simulink model "MX_Gateway"  (Simulink 9.0)
settings: Solver=FixedStepDiscrete  StartTime=0.0  StopTime=100  FixedStep=0.01  RelTol=1e-3
values from neighbouring files (symbols this model uses):
  ACTIVE = Bobcat.Parameter; CreateDefine( ACTIVE                         ,1                          ,'Define' ,'defines.h' )    [defines.m]
  ACTIVE_MODE_ONE = Bobcat.Parameter; CreateDefine( ACTIVE_MODE_ONE                ,19                         ,'Define' ,'defines.h' )    [defines.m]
  AUTHENTICATED = Bobcat.Parameter; CreateDefine( AUTHENTICATED                  ,1                          ,'Define' ,'defines.h' )    [defines.m]
  AUX_EMEA_CONTROLS = Bobcat.Parameter; CreateDefine( AUX_EMEA_CONTROLS  ,1   ,'Define' ,'defines.h' )    [enums.m]
  AUX_FLOW_HIGH = Bobcat.Parameter; CreateDefine( AUX_FLOW_HIGH           ,4  ,'Define' ,'defines.h' )    [enums.m]
  AUX_FLOW_INACTIVE = Bobcat.Parameter; CreateDefine( AUX_FLOW_INACTIVE       ,0  ,'Define' ,'defines.h' )    [enums.m]
  AUX_FLOW_LOW = Bobcat.Parameter; CreateDefine( AUX_FLOW_LOW            ,1  ,'Define' ,'defines.h' )    [enums.m]
  AUX_FLOW_MEDIUM = Bobcat.Parameter; CreateDefine( AUX_FLOW_MEDIUM         ,2  ,'Define' ,'defines.h' )    [enums.m]
  AUX_FLOW_STANDARD = Bobcat.Parameter; CreateDefine( AUX_FLOW_STANDARD       ,3  ,'Define' ,'defines.h' )    [enums.m]
  AUX_NON_FUNCTIONAL = Bobcat.Parameter; CreateDefine( AUX_NON_FUNCTIONAL ,255 ,'Define' ,'defines.h' )    [enums.m]
  BASE_ACTIVE = Bobcat.Parameter; CreateDefine( BASE_ACTIVE                    ,253                        ,'Define' ,'defines.h' )    [defines.m]
  BASE_DETENT = Bobcat.Parameter; CreateDefine( BASE_DETENT                    ,1                          ,'Define' ,'defines.h' )    [defines.m]
  BEEP_NONE = Bobcat.Parameter; CreateDefine( BEEP_NONE                      ,255                        ,'Define' ,'defines.h' )    [defines.m]
  CD_AIR_FILTER_DEBOUNCE_NORMAL = Bobcat.Parameter; CreateAliasCD( CD_AIR_FILTER_DEBOUNCE_NORMAL                        ,uint16(500)                ,'ConstantDataPointer' ,'CONSTANT_DATA' ,'AIR_FILTER_DEBOUNCE_NORMAL')    [cdata.m]    (also in hc12_cdata.m; m4_cdata.m)
  CD_AIR_FILTER_DEBOUNCE_NOT_CONNECTED = Bobcat.Parameter; CreateAliasCD( CD_AIR_FILTER_DEBOUNCE_NOT_CONNECTED                 ,uint16(3000)               ,'ConstantDataPointer' ,'CONSTANT_DATA' ,'AIR_FILTER_DEBOUNCE_NOT_CONNECTED')    [cdata.m]    (also in hc12_cdata.m; m4_cdata.m)
  CD_AIR_FILTER_DEBOUNCE_PLUGGED = Bobcat.Parameter; CreateAliasCD( CD_AIR_FILTER_DEBOUNCE_PLUGGED                       ,uint16(3000)               ,'ConstantDataPointer' ,'CONSTANT_DATA' ,'AIR_FILTER_DEBOUNCE_PLUGGED')    [cdata.m]    (also in hc12_cdata.m; m4_cdata.m)
  CD_AIR_FILTER_FUNCTION = Bobcat.Parameter; CreateAliasCD( CD_AIR_FILTER_FUNCTION                               ,uint8(255)                 ,'ConstantDataPointer' ,'CONSTANT_DATA' ,'AIR_FILTER_FUNCTION')    [cdata.m]    (also in hc12_cdata.m; m4_cdata.m)
  CD_AIR_FILTER_PLUGGED_WEIGHT = Bobcat.Parameter; CreateAliasCD( CD_AIR_FILTER_PLUGGED_WEIGHT                         ,uint8(2)                   ,'ConstantDataPointer' ,'CONSTANT_DATA' ,'AIR_FILTER_PLUGGED_WEIGHT')    [cdata.m]    (also in hc12_cdata.m; m4_cdata.m)
  CD_AIR_FILTER_UNPLUGGED_WEIGHT = Bobcat.Parameter; CreateAliasCD( CD_AIR_FILTER_UNPLUGGED_WEIGHT                       ,uint8(1)                   ,'ConstantDataPointer' ,'CONSTANT_DATA' ,'AIR_FILTER_UNPLUGGED_WEIGHT')    [cdata.m]    (also in hc12_cdata.m; m4_cdata.m)
  CD_AUTOIDLE_ARMBAR_DELAY = Bobcat.Parameter; CreateAliasCD( CD_AUTOIDLE_ARMBAR_DELAY                             ,uint8(2)                   ,'ConstantDataPointer' ,'CONSTANT_DATA' ,'AUTOIDLE_ARMBAR_DELAY')    [cdata.m]    (also in hc12_cdata.m; m4_cdata.m)
  CD_AUTOIDLE_FUNCTION = Bobcat.Parameter; CreateAliasCD( CD_AUTOIDLE_FUNCTION                                 ,uint8(255)                 ,'ConstantDataPointer' ,'CONSTANT_DATA' ,'AUTOIDLE_FUNCTION')    [cdata.m]    (also in hc12_cdata.m; m4_cdata.m)
  CD_AUTOIDLE_IDLE_DELAY = Bobcat.Parameter; CreateAliasCD( CD_AUTOIDLE_IDLE_DELAY                               ,uint8(40)                  ,'ConstantDataPointer' ,'CONSTANT_DATA' ,'AUTOIDLE_IDLE_DELAY')    [cdata.m]    (also in hc12_cdata.m; m4_cdata.m)
  CD_AUTOIDLE_LOW_IDLE_START = Bobcat.Parameter; CreateAliasCD( CD_AUTOIDLE_LOW_IDLE_START                           ,uint8(255)                 ,'ConstantDataPointer' ,'CONSTANT_DATA' ,'AUTOIDLE_LOW_IDLE_START')    [cdata.m]    (also in hc12_cdata.m; m4_cdata.m)
  CD_AUTOIDLE_PRESSURE_OFF = Bobcat.Parameter; CreateAliasCD( CD_AUTOIDLE_PRESSURE_OFF                             ,uint16(300)                ,'ConstantDataPointer' ,'CONSTANT_DATA' ,'AUTOIDLE_PRESSURE_OFF')    [cdata.m]    (also in hc12_cdata.m; m4_cdata.m)
  CD_AUTOIDLE_PRESSURE_ON = Bobcat.Parameter; CreateAliasCD( CD_AUTOIDLE_PRESSURE_ON                              ,uint16(270)                ,'ConstantDataPointer' ,'CONSTANT_DATA' ,'AUTOIDLE_PRESSURE_ON')    [cdata.m]    (also in hc12_cdata.m; m4_cdata.m)
  CD_AUTOSHIFT_FUNCTION = Bobcat.Parameter; CreateAliasCD( CD_AUTOSHIFT_FUNCTION                                ,uint8(255)                 ,'ConstantDataPointer' ,'CONSTANT_DATA' ,'AUTOSHIFT_FUNCTION')    [cdata.m]    (also in hc12_cdata.m; m4_cdata.m)
  CD_AUTOSHIFT_PHIGH1 = Bobcat.Parameter; CreateAliasCD( CD_AUTOSHIFT_PHIGH1                                  ,uint16(205)                ,'ConstantDataPointer' ,'CONSTANT_DATA' ,'AUTOSHIFT_PHIGH1')    [cdata.m]    (also in hc12_cdata.m; m4_cdata.m)
  CD_AUTOSHIFT_PHIGH2 = Bobcat.Parameter; CreateAliasCD( CD_AUTOSHIFT_PHIGH2                                  ,uint16(193)                ,'ConstantDataPointer' ,'CONSTANT_DATA' ,'AUTOSHIFT_PHIGH2')    [cdata.m]    (also in hc12_cdata.m; m4_cdata.m)
  CD_AUTOSHIFT_PHIGH_TEMP1 = Bobcat.Parameter; CreateAliasCD( CD_AUTOSHIFT_PHIGH_TEMP1                             ,int16(70)                  ,'ConstantDataPointer' ,'CONSTANT_DATA' ,'AUTOSHIFT_PHIGH_TEMP1')    [cdata.m]    (also in hc12_cdata.m; m4_cdata.m)
  CD_AUTOSHIFT_PHIGH_TEMP2 = Bobcat.Parameter; CreateAliasCD( CD_AUTOSHIFT_PHIGH_TEMP2                             ,int16(160)                 ,'ConstantDataPointer' ,'CONSTANT_DATA' ,'AUTOSHIFT_PHIGH_TEMP2')    [cdata.m]    (also in hc12_cdata.m; m4_cdata.m)
  CD_AUTOSHIFT_PLOW1 = Bobcat.Parameter; CreateAliasCD( CD_AUTOSHIFT_PLOW1                                   ,uint16(130)                ,'ConstantDataPointer' ,'CONSTANT_DATA' ,'AUTOSHIFT_PLOW1')    [cdata.m]    (also in hc12_cdata.m; m4_cdata.m)
  CD_AUTOSHIFT_PLOW2 = Bobcat.Parameter; CreateAliasCD( CD_AUTOSHIFT_PLOW2                                   ,uint16(100)                ,'ConstantDataPointer' ,'CONSTANT_DATA' ,'AUTOSHIFT_PLOW2')    [cdata.m]    (also in hc12_cdata.m; m4_cdata.m)
  CD_AUTOSHIFT_PLOW_TEMP1 = Bobcat.Parameter; CreateAliasCD( CD_AUTOSHIFT_PLOW_TEMP1                              ,int16(20)                  ,'ConstantDataPointer' ,'CONSTANT_DATA' ,'AUTOSHIFT_PLOW_TEMP1')    [cdata.m]    (also in hc12_cdata.m; m4_cdata.m)
  CD_AUTOSHIFT_PLOW_TEMP2 = Bobcat.Parameter; CreateAliasCD( CD_AUTOSHIFT_PLOW_TEMP2                              ,int16(80)                  ,'ConstantDataPointer' ,'CONSTANT_DATA' ,'AUTOSHIFT_PLOW_TEMP2')    [cdata.m]    (also in hc12_cdata.m; m4_cdata.m)
  CD_AUTOSHIFT_TRAVEL_DELAY = Bobcat.Parameter; CreateAliasCD( CD_AUTOSHIFT_TRAVEL_DELAY                            ,uint8(32)                  ,'ConstantDataPointer' ,'CONSTANT_DATA' ,'AUTOSHIFT_TRAVEL_DELAY')    [cdata.m]    (also in hc12_cdata.m; m4_cdata.m)
  CD_AUX2_CRACKPOINT_CURRENT = Bobcat.Parameter; CreateAliasCD( CD_AUX2_CRACKPOINT_CURRENT                           ,fi(0,0,16,12,'hex','0c28') ,'ConstantDataPointer' ,'CONSTANT_DATA' ,'AUX2_CRACKPOINT_CURRENT')    [cdata.m]    (also in hc12_cdata.m; m4_cdata.m)
  CD_AUX2_FLOW_LOW_FUNCTION = Bobcat.Parameter; CreateAliasCD( CD_AUX2_FLOW_LOW_FUNCTION                            ,uint8(255)                 ,'ConstantDataPointer' ,'CONSTANT_DATA' ,'AUX2_FLOW_LOW_FUNCTION')    [cdata.m]    (also in hc12_cdata.m; m4_cdata.m)
  CD_AUX2_FLOW_MED_FUNCTION = Bobcat.Parameter; CreateAliasCD( CD_AUX2_FLOW_MED_FUNCTION                            ,uint8(255)                 ,'ConstantDataPointer' ,'CONSTANT_DATA' ,'AUX2_FLOW_MED_FUNCTION')    [cdata.m]    (also in hc12_cdata.m; m4_cdata.m)
  CD_AUX2_HI_FLOW_PERCENT = Bobcat.Parameter; CreateAliasCD( CD_AUX2_HI_FLOW_PERCENT                              ,fi(0,0,16,16,'hex','ffff') ,'ConstantDataPointer' ,'CONSTANT_DATA' ,'AUX2_HI_FLOW_PERCENT')    [cdata.m]    (also in hc12_cdata.m; m4_cdata.m)
  CD_AUX2_LOW_FLOW_PERCENT = Bobcat.Parameter; CreateAliasCD( CD_AUX2_LOW_FLOW_PERCENT                             ,fi(0,0,16,16,'hex','7333') ,'ConstantDataPointer' ,'CONSTANT_DATA' ,'AUX2_LOW_FLOW_PERCENT')    [cdata.m]    (also in hc12_cdata.m; m4_cdata.m)
  CD_AUX2_MAX_FLOW_CURRENT = Bobcat.Parameter; CreateAliasCD( CD_AUX2_MAX_FLOW_CURRENT                             ,fi(0,0,16,12,'hex','14cc') ,'ConstantDataPointer' ,'CONSTANT_DATA' ,'AUX2_MAX_FLOW_CURRENT')    [cdata.m]    (also in hc12_cdata.m; m4_cdata.m)
  CD_AUX2_MED_FLOW_PERCENT = Bobcat.Parameter; CreateAliasCD( CD_AUX2_MED_FLOW_PERCENT                             ,fi(0,0,16,16,'hex','9999') ,'ConstantDataPointer' ,'CONSTANT_DATA' ,'AUX2_MED_FLOW_PERCENT')    [cdata.m]    (also in hc12_cdata.m; m4_cdata.m)
  CD_AUX_CRACKPOINT_CURRENT = Bobcat.Parameter; CreateAliasCD( CD_AUX_CRACKPOINT_CURRENT                            ,fi(0,0,16,12,'hex','0000') ,'ConstantDataPointer' ,'CONSTANT_DATA' ,'AUX_CRACKPOINT_CURRENT')    [cdata.m]    (also in hc12_cdata.m; m4_cdata.m)
  CD_AUX_DETENT_FLASH_TIME = Bobcat.Parameter; CreateAliasCD( CD_AUX_DETENT_FLASH_TIME                             ,uint16(72)                 ,'ConstantDataPointer' ,'CONSTANT_DATA' ,'AUX_DETENT_FLASH_TIME')    [cdata.m]    (also in hc12_cdata.m; m4_cdata.m)
  CD_AUX_DETENT_HOLD_TIME = Bobcat.Parameter; CreateAliasCD( CD_AUX_DETENT_HOLD_TIME                              ,uint16(100)                ,'ConstantDataPointer' ,'CONSTANT_DATA' ,'AUX_DETENT_HOLD_TIME')    [cdata.m]    (also in hc12_cdata.m; m4_cdata.m)
  CD_AUX_FULL_ON_AUX1 = Bobcat.Parameter; CreateAliasCD( CD_AUX_FULL_ON_AUX1                                  ,fi(0,0,16,12,'hex','10cd') ,'ConstantDataPointer' ,'CONSTANT_DATA' ,'AUX_FULL_ON_AUX1')    [cdata.m]    (also in hc12_cdata.m; m4_cdata.m)
  CD_AUX_FULL_ON_AUX2 = Bobcat.Parameter; CreateAliasCD( CD_AUX_FULL_ON_AUX2                                  ,fi(0,0,16,12,'hex','139d') ,'ConstantDataPointer' ,'CONSTANT_DATA' ,'AUX_FULL_ON_AUX2')    [cdata.m]    (also in hc12_cdata.m; m4_cdata.m)
  CD_AUX_FULL_ON_AUX3 = Bobcat.Parameter; CreateAliasCD( CD_AUX_FULL_ON_AUX3                                  ,fi(0,0,16,12,'hex','17d7') ,'ConstantDataPointer' ,'CONSTANT_DATA' ,'AUX_FULL_ON_AUX3')    [cdata.m]    (also in hc12_cdata.m; m4_cdata.m)
  CD_AUX_FUNCTION = Bobcat.Parameter; CreateAliasCD( CD_AUX_FUNCTION                                      ,uint8(0)                   ,'ConstantDataPointer' ,'CONSTANT_DATA' ,'AUX_FUNCTION')    [cdata.m]    (also in hc12_cdata.m; m4_cdata.m)
  CD_AUX_RATE_LIMIT_CRACKPOINT = Bobcat.Parameter; CreateAliasCD( CD_AUX_RATE_LIMIT_CRACKPOINT                         ,fi(0,0,16,12,'hex','0800') ,'ConstantDataPointer' ,'CONSTANT_DATA' ,'AUX_RATE_LIMIT_CRACKPOINT')    [cdata.m]    (also in hc12_cdata.m; m4_cdata.m)
  CD_AUX_RATE_LIMIT_CRACKPOINT_RATE = Bobcat.Parameter; CreateAliasCD( CD_AUX_RATE_LIMIT_CRACKPOINT_RATE                    ,fi(0,1,32,12,'int',102400) ,'ConstantDataPointer' ,'CONSTANT_DATA' ,'AUX_RATE_LIMIT_CRACKPOINT_RATE')    [cdata.m]    (also in hc12_cdata.m; m4_cdata.m)
  CD_AUX_RATE_LIMIT_FUNCTION = Bobcat.Parameter; CreateAliasCD( CD_AUX_RATE_LIMIT_FUNCTION                           ,uint8(255)                 ,'ConstantDataPointer' ,'CONSTANT_DATA' ,'AUX_RATE_LIMIT_FUNCTION')    [cdata.m]    (also in hc12_cdata.m; m4_cdata.m)
  CD_AUX_RATE_LIMIT_MAX_ENG_SPD = Bobcat.Parameter; CreateAliasCD( CD_AUX_RATE_LIMIT_MAX_ENG_SPD                        ,uint16(1600)               ,'ConstantDataPointer' ,'CONSTANT_DATA' ,'AUX_RATE_LIMIT_MAX_ENG_SPD')    [cdata.m]    (also in hc12_cdata.m; m4_cdata.m)
  CD_AUX_RATE_LIMIT_MIN_ENG_SPD = Bobcat.Parameter; CreateAliasCD( CD_AUX_RATE_LIMIT_MIN_ENG_SPD                        ,uint16(100)                ,'ConstantDataPointer' ,'CONSTANT_DATA' ,'AUX_RATE_LIMIT_MIN_ENG_SPD')    [cdata.m]    (also in hc12_cdata.m; m4_cdata.m)
  CD_AUX_RATE_LIMIT_NORMAL_RATE = Bobcat.Parameter; CreateAliasCD( CD_AUX_RATE_LIMIT_NORMAL_RATE                        ,fi(0,1,32,12,'int',2048)   ,'ConstantDataPointer' ,'CONSTANT_DATA' ,'AUX_RATE_LIMIT_NORMAL_RATE')    [cdata.m]    (also in hc12_cdata.m; m4_cdata.m)
  CD_AUXPOT_CAL_DEADBAND_HIGH = Bobcat.Parameter; CreateAliasCD( CD_AUXPOT_CAL_DEADBAND_HIGH                          ,uint16(574)                ,'ConstantDataPointer' ,'CONSTANT_DATA' ,'AUXPOT_CAL_DEADBAND_HIGH')    [cdata.m]    (also in hc12_cdata.m; m4_cdata.m)
  CD_AUXPOT_CAL_DEADBAND_LOW = Bobcat.Parameter; CreateAliasCD( CD_AUXPOT_CAL_DEADBAND_LOW                           ,uint16(424)                ,'ConstantDataPointer' ,'CONSTANT_DATA' ,'AUXPOT_CAL_DEADBAND_LOW')    [cdata.m]    (also in hc12_cdata.m; m4_cdata.m)
  CD_AUXPOT_CENTER_RATIO = Bobcat.Parameter; CreateAliasCD( CD_AUXPOT_CENTER_RATIO                               ,uint16(500)                ,'ConstantDataPointer' ,'CONSTANT_DATA' ,'AUXPOT_CENTER_RATIO')    [cdata.m]    (also in hc12_cdata.m; m4_cdata.m)
  CD_AUXPOT_DEADBAND = Bobcat.Parameter; CreateAliasCD( CD_AUXPOT_DEADBAND                                   ,uint8(38)                  ,'ConstantDataPointer' ,'CONSTANT_DATA' ,'AUXPOT_DEADBAND')    [cdata.m]    (also in hc12_cdata.m; m4_cdata.m)
  CD_AUXPOT_FUNCTION = Bobcat.Parameter; CreateAliasCD( CD_AUXPOT_FUNCTION                                   ,uint8(0)                   ,'ConstantDataPointer' ,'CONSTANT_DATA' ,'AUXPOT_FUNCTION')    [cdata.m]    (also in hc12_cdata.m; m4_cdata.m)
  CD_AUXPOT_RATIO_GAIN = Bobcat.Parameter; CreateAliasCD( CD_AUXPOT_RATIO_GAIN                                 ,uint16(1000)               ,'ConstantDataPointer' ,'CONSTANT_DATA' ,'AUXPOT_RATIO_GAIN')    [cdata.m]    (also in hc12_cdata.m; m4_cdata.m)
  CD_CAN_THROTTLE_FUNCTION = Bobcat.Parameter; CreateAliasCD( CD_CAN_THROTTLE_FUNCTION                             ,uint8(255)                 ,'ConstantDataPointer' ,'CONSTANT_DATA' ,'CAN_THROTTLE_FUNCTION')    [cdata.m]    (also in hc12_cdata.m; m4_cdata.m)
  CD_CAN_THROTTLE_REVERSE = Bobcat.Parameter; CreateAliasCD( CD_CAN_THROTTLE_REVERSE                              ,uint8(255)                 ,'ConstantDataPointer' ,'CONSTANT_DATA' ,'CAN_THROTTLE_REVERSE')    [cdata.m]    (also in hc12_cdata.m; m4_cdata.m)
  CD_CONSOLE_DEBOUNCE_ABSENT = Bobcat.Parameter; CreateAliasCD( CD_CONSOLE_DEBOUNCE_ABSENT                           ,uint16(12)                 ,'ConstantDataPointer' ,'CONSTANT_DATA' ,'CONSOLE_DEBOUNCE_ABSENT')    [cdata.m]    (also in hc12_cdata.m; m4_cdata.m)
  CD_CONSOLE_DEBOUNCE_OORH = Bobcat.Parameter; CreateAliasCD( CD_CONSOLE_DEBOUNCE_OORH                             ,uint16(12)                 ,'ConstantDataPointer' ,'CONSTANT_DATA' ,'CONSOLE_DEBOUNCE_OORH')    [cdata.m]    (also in hc12_cdata.m; m4_cdata.m)
  CD_CONSOLE_DEBOUNCE_OORL = Bobcat.Parameter; CreateAliasCD( CD_CONSOLE_DEBOUNCE_OORL                             ,uint16(12)                 ,'ConstantDataPointer' ,'CONSTANT_DATA' ,'CONSOLE_DEBOUNCE_OORL')    [cdata.m]    (also in hc12_cdata.m; m4_cdata.m)
  CD_CONSOLE_DEBOUNCE_PRESENT = Bobcat.Parameter; CreateAliasCD( CD_CONSOLE_DEBOUNCE_PRESENT                          ,uint16(12)                 ,'ConstantDataPointer' ,'CONSTANT_DATA' ,'CONSOLE_DEBOUNCE_PRESENT')    [cdata.m]    (also in hc12_cdata.m; m4_cdata.m)
  CD_CONSOLE_FUNCTION = Bobcat.Parameter; CreateAliasCD( CD_CONSOLE_FUNCTION                                  ,uint8(0)                   ,'ConstantDataPointer' ,'CONSTANT_DATA' ,'CONSOLE_FUNCTION')    [cdata.m]    (also in hc12_cdata.m; m4_cdata.m)
  CD_CONSOLE_OORH = Bobcat.Parameter; CreateAliasCD( CD_CONSOLE_OORH                                      ,uint16(740)                ,'ConstantDataPointer' ,'CONSTANT_DATA' ,'CONSOLE_OORH')    [cdata.m]    (also in hc12_cdata.m; m4_cdata.m)
  CD_CONSOLE_OORL = Bobcat.Parameter; CreateAliasCD( CD_CONSOLE_OORL                                      ,uint16(10)                 ,'ConstantDataPointer' ,'CONSTANT_DATA' ,'CONSOLE_OORL')    [cdata.m]    (also in hc12_cdata.m; m4_cdata.m)
  CD_CONSOLE_PRESENT_HIGH = Bobcat.Parameter; CreateAliasCD( CD_CONSOLE_PRESENT_HIGH                              ,uint16(515)                ,'ConstantDataPointer' ,'CONSTANT_DATA' ,'CONSOLE_PRESENT_HIGH')    [cdata.m]    (also in hc12_cdata.m; m4_cdata.m)
  CD_CONSOLE_PRESENT_LOW = Bobcat.Parameter; CreateAliasCD( CD_CONSOLE_PRESENT_LOW                               ,uint16(375)                ,'ConstantDataPointer' ,'CONSTANT_DATA' ,'CONSOLE_PRESENT_LOW')    [cdata.m]    (also in hc12_cdata.m; m4_cdata.m)
  CD_DELUXE_GEN5_5_FUNCTION = Bobcat.Parameter; CreateAliasCD( CD_DELUXE_GEN5_5_FUNCTION                            ,uint8(255)                 ,'ConstantDataPointer' ,'CONSTANT_DATA' ,'DELUXE_GEN5_5_FUNCTION')    [cdata.m]    (also in hc12_cdata.m; m4_cdata.m)
  CD_DELUXE_GEN5_FUNCTION = Bobcat.Parameter; CreateAliasCD( CD_DELUXE_GEN5_FUNCTION                              ,uint8(255)                 ,'ConstantDataPointer' ,'CONSTANT_DATA' ,'DELUXE_GEN5_FUNCTION')    [cdata.m]    (also in hc12_cdata.m; m4_cdata.m)
  CD_DERATE_ENGINE_SPEED_DEBOUNCE_HIGH = Bobcat.Parameter; CreateAliasCD( CD_DERATE_ENGINE_SPEED_DEBOUNCE_HIGH                 ,uint16(200)                ,'ConstantDataPointer' ,'CONSTANT_DATA' ,'DERATE_ENGINE_SPEED_DEBOUNCE_HIGH')    [cdata.m]    (also in hc12_cdata.m; m4_cdata.m)
  CD_DERATE_ENGINE_SPEED_DEBOUNCE_SHUTDOWN = Bobcat.Parameter; CreateAliasCD( CD_DERATE_ENGINE_SPEED_DEBOUNCE_SHUTDOWN             ,uint16(1000)               ,'ConstantDataPointer' ,'CONSTANT_DATA' ,'DERATE_ENGINE_SPEED_DEBOUNCE_SHUTDOWN')    [cdata.m]    (also in hc12_cdata.m; m4_cdata.m)
  CD_DERATE_ENGINE_SPEED_HIGH_OFFSET = Bobcat.Parameter; CreateAliasCD( CD_DERATE_ENGINE_SPEED_HIGH_OFFSET                   ,uint16(200)                ,'ConstantDataPointer' ,'CONSTANT_DATA' ,'DERATE_ENGINE_SPEED_HIGH_OFFSET')    [cdata.m]    (also in hc12_cdata.m; m4_cdata.m)
  CD_DERATE_METHOD = Bobcat.Parameter; CreateAliasCD( CD_DERATE_METHOD                                     ,uint8(0)                   ,'ConstantDataPointer' ,'CONSTANT_DATA' ,'DERATE_METHOD')    [cdata.m]    (also in hc12_cdata.m; m4_cdata.m)
  CD_DUMP_VALVE_FUNCTION = Bobcat.Parameter; CreateAliasCD( CD_DUMP_VALVE_FUNCTION                               ,uint8(255)                 ,'ConstantDataPointer' ,'CONSTANT_DATA' ,'DUMP_VALVE_FUNCTION')    [cdata.m]    (also in hc12_cdata.m; m4_cdata.m)
  CD_DUMP_VALVE_ON_CURRENT = Bobcat.Parameter; CreateAliasCD( CD_DUMP_VALVE_ON_CURRENT                             ,fi(0,0,16,12,'hex','ffff') ,'ConstantDataPointer' ,'CONSTANT_DATA' ,'DUMP_VALVE_ON_CURRENT')    [cdata.m]    (also in hc12_cdata.m; m4_cdata.m)
  CD_ECO_MODE_THROTTLE_REDUCTION = Bobcat.Parameter; CreateAliasCD( CD_ECO_MODE_THROTTLE_REDUCTION                       ,uint16(850)                ,'ConstantDataPointer' ,'CONSTANT_DATA' ,'ECO_MODE_THROTTLE_REDUCTION')    [cdata.m]    (also in hc12_cdata.m; m4_cdata.m)
  CD_ECT_FILTER_GAIN = Bobcat.Parameter; CreateAliasCD( CD_ECT_FILTER_GAIN                                   ,uint8(6)                   ,'ConstantDataPointer' ,'CONSTANT_DATA' ,'ECT_FILTER_GAIN')    [cdata.m]    (also in hc12_cdata.m; m4_cdata.m)
  CD_ECU_FUNCTION = Bobcat.Parameter; CreateAliasCD( CD_ECU_FUNCTION                                      ,uint8(255)                 ,'ConstantDataPointer' ,'CONSTANT_DATA' ,'ECU_FUNCTION')    [cdata.m]    (also in hc12_cdata.m; m4_cdata.m)
  CD_ECU_HOLD_DEBOUNCE_ERROR_ON = Bobcat.Parameter; CreateAliasCD( CD_ECU_HOLD_DEBOUNCE_ERROR_ON                        ,uint16(20)                 ,'ConstantDataPointer' ,'CONSTANT_DATA' ,'ECU_HOLD_DEBOUNCE_ERROR_ON')    [cdata.m]    (also in hc12_cdata.m; m4_cdata.m)
  CD_EMEA_CONTROLS_HOLD_TIME = Bobcat.Parameter; CreateAliasCD( CD_EMEA_CONTROLS_HOLD_TIME                           ,uint16(30)                 ,'ConstantDataPointer' ,'CONSTANT_DATA' ,'EMEA_CONTROLS_HOLD_TIME')    [cdata.m]    (also in hc12_cdata.m; m4_cdata.m)
  CD_EMEA_CONTROLS_OPTION_A = Bobcat.Parameter; CreateAliasCD( CD_EMEA_CONTROLS_OPTION_A                            ,uint8(255)                 ,'ConstantDataPointer' ,'CONSTANT_DATA' ,'EMEA_CONTROLS_OPTION_A')    [cdata.m]    (also in hc12_cdata.m; m4_cdata.m)
  CD_EMEA_CONTROLS_OPTION_C = Bobcat.Parameter; CreateAliasCD( CD_EMEA_CONTROLS_OPTION_C                            ,uint8(255)                 ,'ConstantDataPointer' ,'CONSTANT_DATA' ,'EMEA_CONTROLS_OPTION_C')    [cdata.m]    (also in hc12_cdata.m; m4_cdata.m)
  CD_EMEA_CONTROLS_REVERSE = Bobcat.Parameter; CreateAliasCD( CD_EMEA_CONTROLS_REVERSE                             ,uint8(255)                 ,'ConstantDataPointer' ,'CONSTANT_DATA' ,'EMEA_CONTROLS_REVERSE')    [cdata.m]    (also in hc12_cdata.m; m4_cdata.m)
  CD_EMEA_ELECTRIC_VERSION = Bobcat.Parameter; CreateAliasCD( CD_EMEA_ELECTRIC_VERSION                             ,uint8(0)                   ,'ConstantDataPointer' ,'CONSTANT_DATA' ,'EMEA_ELECTRIC_VERSION')    [cdata.m]    (also in hc12_cdata.m; m4_cdata.m)
  CD_EMEA_POT_CAL_DEADBAND_HIGH = Bobcat.Parameter; CreateAliasCD( CD_EMEA_POT_CAL_DEADBAND_HIGH                        ,uint16(574)                ,'ConstantDataPointer' ,'CONSTANT_DATA' ,'EMEA_POT_CAL_DEADBAND_HIGH')    [cdata.m]    (also in hc12_cdata.m; m4_cdata.m)
  CD_EMEA_POT_CAL_DEADBAND_LOW = Bobcat.Parameter; CreateAliasCD( CD_EMEA_POT_CAL_DEADBAND_LOW                         ,uint16(424)                ,'ConstantDataPointer' ,'CONSTANT_DATA' ,'EMEA_POT_CAL_DEADBAND_LOW')    [cdata.m]    (also in hc12_cdata.m; m4_cdata.m)
  CD_ENGINE_RPM_FACTOR = Bobcat.Parameter; CreateAliasCD( CD_ENGINE_RPM_FACTOR                                 ,uint8(35)                  ,'ConstantDataPointer' ,'CONSTANT_DATA' ,'ENGINE_RPM_FACTOR')    [cdata.m]    (also in hc12_cdata.m; m4_cdata.m)
  CD_ENGINE_SPEED_FILTER_FAST_VALUE = Bobcat.Parameter; CreateAliasCD( CD_ENGINE_SPEED_FILTER_FAST_VALUE                    ,uint8(1)                   ,'ConstantDataPointer' ,'CONSTANT_DATA' ,'ENGINE_SPEED_FILTER_FAST_VALUE')    [cdata.m]    (also in hc12_cdata.m; m4_cdata.m)
  CD_ENGINE_SPEED_FILTER_SLOW_VALUE = Bobcat.Parameter; CreateAliasCD( CD_ENGINE_SPEED_FILTER_SLOW_VALUE                    ,uint8(4)                   ,'ConstantDataPointer' ,'CONSTANT_DATA' ,'ENGINE_SPEED_FILTER_SLOW_VALUE')    [cdata.m]    (also in hc12_cdata.m; m4_cdata.m)
  CD_ENGINE_SPEED_RUNNING = Bobcat.Parameter; CreateAliasCD( CD_ENGINE_SPEED_RUNNING                              ,uint16(800)                ,'ConstantDataPointer' ,'CONSTANT_DATA' ,'ENGINE_SPEED_RUNNING')    [cdata.m]    (also in hc12_cdata.m; m4_cdata.m)
  CD_ENGINE_START_DELAY_DEBOUNCE_RPM_NO_SIGNAL = Bobcat.Parameter; CreateAliasCD( CD_ENGINE_START_DELAY_DEBOUNCE_RPM_NO_SIGNAL         ,uint16(0)                  ,'ConstantDataPointer' ,'CONSTANT_DATA' ,'ENGINE_START_DELAY_DEBOUNCE_RPM_NO_SIGNAL')    [cdata.m]    (also in hc12_cdata.m; m4_cdata.m)
  CD_ENGINE_START_DELAY_OFF_TIME = Bobcat.Parameter; CreateAliasCD( CD_ENGINE_START_DELAY_OFF_TIME                       ,uint16(0)                  ,'ConstantDataPointer' ,'CONSTANT_DATA' ,'ENGINE_START_DELAY_OFF_TIME')    [cdata.m]    (also in hc12_cdata.m; m4_cdata.m)
  CD_ENGINE_START_DELAY_RPM_NO_SIGNAL = Bobcat.Parameter; CreateAliasCD( CD_ENGINE_START_DELAY_RPM_NO_SIGNAL                  ,uint16(0)                  ,'ConstantDataPointer' ,'CONSTANT_DATA' ,'ENGINE_START_DELAY_RPM_NO_SIGNAL')    [cdata.m]    (also in hc12_cdata.m; m4_cdata.m)
  CD_EOP_DEBOUNCE_EXTREMELY_LOW = Bobcat.Parameter; CreateAliasCD( CD_EOP_DEBOUNCE_EXTREMELY_LOW                        ,uint16(50)                 ,'ConstantDataPointer' ,'CONSTANT_DATA' ,'EOP_DEBOUNCE_EXTREMELY_LOW')    [cdata.m]    (also in hc12_cdata.m; m4_cdata.m)
  CD_EOP_DEBOUNCE_NORMAL = Bobcat.Parameter; CreateAliasCD( CD_EOP_DEBOUNCE_NORMAL                               ,uint16(50)                 ,'ConstantDataPointer' ,'CONSTANT_DATA' ,'EOP_DEBOUNCE_NORMAL')    [cdata.m]    (also in hc12_cdata.m; m4_cdata.m)
  CD_EOP_DEBOUNCE_SHUTDOWN = Bobcat.Parameter; CreateAliasCD( CD_EOP_DEBOUNCE_SHUTDOWN                             ,uint16(100)                ,'ConstantDataPointer' ,'CONSTANT_DATA' ,'EOP_DEBOUNCE_SHUTDOWN')    [cdata.m]    (also in hc12_cdata.m; m4_cdata.m)
  CD_EOP_FILTER_VALUE = Bobcat.Parameter; CreateAliasCD( CD_EOP_FILTER_VALUE                                  ,uint8(3)                   ,'ConstantDataPointer' ,'CONSTANT_DATA' ,'EOP_FILTER_VALUE')    [cdata.m]    (also in hc12_cdata.m; m4_cdata.m)
  CD_EOP_FUNCTION = Bobcat.Parameter; CreateAliasCD( CD_EOP_FUNCTION                                      ,uint8(1)                   ,'ConstantDataPointer' ,'CONSTANT_DATA' ,'EOP_FUNCTION')    [cdata.m]    (also in hc12_cdata.m; m4_cdata.m)
  CD_EOP_SWITCH_GAUGE_VALUE = Bobcat.Parameter; CreateAliasCD( CD_EOP_SWITCH_GAUGE_VALUE                            ,uint16(125)                ,'ConstantDataPointer' ,'CONSTANT_DATA' ,'EOP_SWITCH_GAUGE_VALUE')    [cdata.m]    (also in hc12_cdata.m; m4_cdata.m)
  CD_EOP_SWITCH_LOW = Bobcat.Parameter; CreateAliasCD( CD_EOP_SWITCH_LOW                                    ,uint16(50)                 ,'ConstantDataPointer' ,'CONSTANT_DATA' ,'EOP_SWITCH_LOW')    [cdata.m]    (also in hc12_cdata.m; m4_cdata.m)
  CD_EOP_SWITCH_PRESSURE_VALUE = Bobcat.Parameter; CreateAliasCD( CD_EOP_SWITCH_PRESSURE_VALUE                         ,uint16(40)                 ,'ConstantDataPointer' ,'CONSTANT_DATA' ,'EOP_SWITCH_PRESSURE_VALUE')    [cdata.m]    (also in hc12_cdata.m; m4_cdata.m)
  CD_EOT_DEBOUNCE_EXTREMELY_LOW = Bobcat.Parameter; CreateAliasCD( CD_EOT_DEBOUNCE_EXTREMELY_LOW                        ,uint16(2)                  ,'ConstantDataPointer' ,'CONSTANT_DATA' ,'EOT_DEBOUNCE_EXTREMELY_LOW')    [cdata.m]    (also in hc12_cdata.m; m4_cdata.m)
  CD_EOT_DEBOUNCE_LOW = Bobcat.Parameter; CreateAliasCD( CD_EOT_DEBOUNCE_LOW                                  ,uint16(2)                  ,'ConstantDataPointer' ,'CONSTANT_DATA' ,'EOT_DEBOUNCE_LOW')    [cdata.m]    (also in hc12_cdata.m; m4_cdata.m)
  CD_EOT_DEBOUNCE_NORMAL = Bobcat.Parameter; CreateAliasCD( CD_EOT_DEBOUNCE_NORMAL                               ,uint16(2)                  ,'ConstantDataPointer' ,'CONSTANT_DATA' ,'EOT_DEBOUNCE_NORMAL')    [cdata.m]    (also in hc12_cdata.m; m4_cdata.m)
  CD_EOT_EXTREMELY_LOW = Bobcat.Parameter; CreateAliasCD( CD_EOT_EXTREMELY_LOW                                 ,uint16(81)                 ,'ConstantDataPointer' ,'CONSTANT_DATA' ,'EOT_EXTREMELY_LOW')    [cdata.m]    (also in hc12_cdata.m; m4_cdata.m)
  CD_EOT_FUNCTION = Bobcat.Parameter; CreateAliasCD( CD_EOT_FUNCTION                                      ,uint8(255)                 ,'ConstantDataPointer' ,'CONSTANT_DATA' ,'EOT_FUNCTION')    [cdata.m]    (also in hc12_cdata.m; m4_cdata.m)
  CD_EOT_LOW = Bobcat.Parameter; CreateAliasCD( CD_EOT_LOW                                           ,uint16(90)                 ,'ConstantDataPointer' ,'CONSTANT_DATA' ,'EOT_LOW')    [cdata.m]    (also in hc12_cdata.m; m4_cdata.m)
  CD_EOT_TEMPERATURE_HYSTERESIS = Bobcat.Parameter; CreateAliasCD( CD_EOT_TEMPERATURE_HYSTERESIS                        ,uint16(2)                  ,'ConstantDataPointer' ,'CONSTANT_DATA' ,'EOT_TEMPERATURE_HYSTERESIS')    [cdata.m]    (also in hc12_cdata.m; m4_cdata.m)
  CD_FAST_TRAVEL_FUNCTION = Bobcat.Parameter; CreateAliasCD( CD_FAST_TRAVEL_FUNCTION                              ,uint8(255)                 ,'ConstantDataPointer' ,'CONSTANT_DATA' ,'FAST_TRAVEL_FUNCTION')    [cdata.m]    (also in hc12_cdata.m; m4_cdata.m)
  CD_FAST_TRAVEL_THROTTLE_DELAY = Bobcat.Parameter; CreateAliasCD( CD_FAST_TRAVEL_THROTTLE_DELAY                        ,uint16(10)                 ,'ConstantDataPointer' ,'CONSTANT_DATA' ,'FAST_TRAVEL_THROTTLE_DELAY')    [cdata.m]    (also in hc12_cdata.m; m4_cdata.m)
  CD_FAST_TRAVEL_THROTTLE_LIMIT = Bobcat.Parameter; CreateAliasCD( CD_FAST_TRAVEL_THROTTLE_LIMIT                        ,uint16(1000)               ,'ConstantDataPointer' ,'CONSTANT_DATA' ,'FAST_TRAVEL_THROTTLE_LIMIT')    [cdata.m]    (also in hc12_cdata.m; m4_cdata.m)
  CD_FLYWHEEL_TEETH = Bobcat.Parameter; CreateAliasCD( CD_FLYWHEEL_TEETH                                    ,uint8(83)                  ,'ConstantDataPointer' ,'CONSTANT_DATA' ,'FLYWHEEL_TEETH')    [cdata.m]    (also in hc12_cdata.m; m4_cdata.m)
  CD_FUEL_LEVEL_FILTER_FAST_VALUE = Bobcat.Parameter; CreateAliasCD( CD_FUEL_LEVEL_FILTER_FAST_VALUE                      ,uint8(5)                   ,'ConstantDataPointer' ,'CONSTANT_DATA' ,'FUEL_LEVEL_FILTER_FAST_VALUE')    [cdata.m]    (also in hc12_cdata.m; m4_cdata.m)
  CD_FUEL_LEVEL_FILTER_SLOW_VALUE = Bobcat.Parameter; CreateAliasCD( CD_FUEL_LEVEL_FILTER_SLOW_VALUE                      ,uint8(10)                  ,'ConstantDataPointer' ,'CONSTANT_DATA' ,'FUEL_LEVEL_FILTER_SLOW_VALUE')    [cdata.m]    (also in hc12_cdata.m; m4_cdata.m)
  CD_FUEL_PRIMARY_FEEDBACK_FUNCTION = Bobcat.Parameter; CreateAliasCD( CD_FUEL_PRIMARY_FEEDBACK_FUNCTION                    ,uint8(0)                   ,'ConstantDataPointer' ,'CONSTANT_DATA' ,'FUEL_PRIMARY_FEEDBACK_FUNCTION')    [cdata.m]    (also in hc12_cdata.m; m4_cdata.m)
  ... (+246 more)
undefined symbols (not defined in the file or in its folder): sigbuilder_block
undefined symbols (not defined in the file): CD_FUEL_RESOLUTION_TIMER
libraries: simulink x186, MX_Library x107
(part 18 of 48)

== system MX_Gateway/MX_Gtwy_Control/System/AutoIdle ==
  psi_LoadSense : Inport
  state_LoadSense : Inport  Port=2
  state_Console : Inport  Port=3
  percent_Throttle : Inport  Port=4
  button_AutoIdle : Inport  Port=5
  state_Engine : Inport  Port=6
  state_System : Inport  Port=7
  flag_InitEERead : Inport  Port=8
  dlx_G55_AutoIdleTime : Inport  Port=9
  dlx_G55_AutoIdleEnable : Inport  Port=10
  AutoIdleTime : Truth Table  TreatAsAtomicUnit=on
  Auto_Idle_Enable : Subsystem  RequirementInfo={} %GIDa_1bcaf31e_eccf_4f63_b0fa_03591ea49f6a  TreatAsAtomicUnit=on  SystemSampleTime=srate100  RTWFcnName=mod_engine_control  RTWFileName=mod_System
      settings: RTWSystemCode=Nonreusable function  RTWFcnNameOpts=Use subsystem name  RTWFileNameOpts=User specified
  Auto_Idle_Function : Subsystem
  Compare To Constant : simulink/Logic and Bit Operations/Compare To Constant  relop===  const=SYS_NORMAL  OutDataTypeStr=boolean
  cmd_AutoIdle : Outport  OutputWhenDisabled=reset
  state_AutoIdleEnable : Outport  Port=2  OutputWhenDisabled=reset
  state_Idle : Outport  Port=3
  wires:
  psi_LoadSense/1 -> Auto_Idle_Function/1(psi_LoadSense)
  state_Console/1 -> Auto_Idle_Function/2(state_Console)
  state_Engine/1 -> Auto_Idle_Function/4(state_Engine)
  Auto_Idle_Function/1(cmd_AutoIdle) -> cmd_AutoIdle/1
  percent_Throttle/1 -> Auto_Idle_Function/5(percent_Throttle)
  state_System/1 -> Compare To Constant/1
  Compare To Constant/1 -> Auto_Idle_Function/Enable
  Auto_Idle_Enable/1(state_AutoIdleEnable) -> [state_AutoIdleEnable], state_AutoIdleEnable/1
  state_LoadSense/1 -> Auto_Idle_Enable/2(state_LoadSense)
  button_AutoIdle/1 -> Auto_Idle_Enable/1(button_AutoIdle)
  [state_AutoIdleEnable] -> Auto_Idle_Function/3(state_AutoIdleEnable)
  Auto_Idle_Function/2(state_Idle) -> state_Idle/1
  flag_InitEERead/1 -> Auto_Idle_Enable/3(flag_InitEERead)
  dlx_G55_AutoIdleTime/1 -> AutoIdleTime/1(dlx_G55_AutoIdleTime)
  AutoIdleTime/1(AutoIdleTime) -> Auto_Idle_Function/6(AutoIdleTime)
  dlx_G55_AutoIdleEnable/1 -> Auto_Idle_Enable/4(dlx_G55_AutoIdleEnable)

== system MX_Gateway/MX_Gtwy_Control/System/Aux_Enable ==
  aux_DebounceButton : Inport
  state_AuxPot : Inport  Port=2
  state_console : Inport  Port=3
  aux_DetentHold : Inport  Port=4
  state_Buttons : Inport  Port=5
  button_AuxReleaseAck : Inport  Port=6
  button_AuxReleaseBack : Inport  Port=7
  button_AuxReleaseExit : Inport  Port=8
  button_Aux : Inport  Port=9
  state_RightPot : Inport  Port=10
  state_EngineSpeed : Inport  Port=11
  din_Detent : Inport  Port=12
  state_System : Inport  Port=13
  flag_InitEERead : Inport  Port=14
  state_ST_CAN : Inport  Port=15
  can_AuxEnable_ST : Inport  Port=16
  state_ACD_CAN : Inport  Port=17
  can_AuxEnable_ACD : Inport  Port=18
  state_Engine : Inport  Port=19
  change_OffsetSelectSw : Inport  Port=20
  mode_RightControl : Inport  Port=21
  aux_Inhibit : Inport  Port=22
  Aux_Control : Stateflow chart  RequirementInfo="{}  %GIDa_112d2c1f_2f82_49d8_b987_6745627d4a42"  TreatAsAtomicUnit=on
  DetectFallingEdge : simulink/Logic and Bit Operations/Detect Decrease  vinit=0.0  InputProcessing=Inherited  OutDataTypeStr=uint8
  DetectRisingEdge1 : simulink/Logic and Bit Operations/Detect Increase  vinit=0.0  InputProcessing=Inherited  OutDataTypeStr=uint8
  DetectRisingEdge2 : simulink/Logic and Bit Operations/Detect Increase  vinit=0.0  InputProcessing=Inherited  OutDataTypeStr=uint8
  state_AuxEnable : Outport
  state_Detent : Outport  Port=2
  aux_Flow : Outport  Port=3
  state_DetentEnable : Outport  Port=4
  cmd_AuxRelease : Outport  Port=5
  state_AuxRelease : Outport  Port=6
  wires:
  din_Detent/1 -> DetectRisingEdge1/1
  state_Engine/1 -> Aux_Control/3(state_Engine)
  Aux_Control/1(state_AuxEnable) -> state_AuxEnable/1
  Aux_Control/2(state_Detent) -> state_Detent/1
  state_console/1 -> Aux_Control/4(state_Console)
  DetectRisingEdge1/1 -> Aux_Control/1(DetentRisingEdge)
  Aux_Control/3(aux_Flow) -> aux_Flow/1
  aux_DebounceButton/1 -> Aux_Control/5(aux_DebounceButton)
  Aux_Control/4(state_DetentEnable) -> state_DetentEnable/1
  aux_DetentHold/1 -> DetectRisingEdge2/1
  DetectRisingEdge2/1 -> Aux_Control/7(aux_DetentHold)
  state_Buttons/1 -> Aux_Control/8(state_Buttons)
  state_System/1 -> Aux_Control/6(state_System)
  flag_InitEERead/1 -> Aux_Control/9(flag_InitEERead)
  can_AuxEnable_ST/1 -> Aux_Control/10(can_AuxEnable_ST)
  can_AuxEnable_ACD/1 -> Aux_Control/11(can_AuxEnable_ACD)
  state_ST_CAN/1 -> Aux_Control/12(state_ST_CAN)
  state_ACD_CAN/1 -> Aux_Control/13(state_ACD_CAN)
  Aux_Control/5(cmd_AuxRelease) -> cmd_AuxRelease/1
  button_AuxReleaseAck/1 -> Aux_Control/14(button_AuxReleaseAck)
  button_AuxReleaseBack/1 -> Aux_Control/15(button_AuxReleaseBack)
  button_AuxReleaseExit/1 -> Aux_Control/16(button_AuxReleaseExit)
  button_Aux/1 -> DetectFallingEdge/1
  DetectFallingEdge/1 -> Aux_Control/17(button_Aux)
  Aux_Control/6(state_AuxRelease) -> state_AuxRelease/1
  state_RightPot/1 -> Aux_Control/18(state_RightPot)
  change_OffsetSelectSw/1 -> Aux_Control/19(change_OffsetSelectSw)
  mode_RightControl/1 -> Aux_Control/20(mode_RightControl)
  aux_Inhibit/1 -> Aux_Control/21(aux_Inhibit)
  state_EngineSpeed/1 -> Aux_Control/22(state_EngineSpeed)
  state_AuxPot/1 -> Aux_Control/2(state_AuxPot)

== system MX_Gateway/MX_Gtwy_Control/System/CAN_Throttle ==
  state_System : Inport
  fdbk_CANThrottle : Inport  Port=2
  flag_InitEERead : Inport  Port=3
  pos_Throttle : Inport  Port=4
  state_Engine : Inport  Port=5
  state_CommThrottle : Inport  Port=6
  cmd_Throttle : Inport  Port=7
  CAN_Throttle : Subsystem  TreatAsAtomicUnit=on
  CompareToConstant1 : simulink/Logic and Bit Operations/Compare To Constant  relop=~=  const=NON_FUNCTIONAL  OutDataTypeStr=boolean  ZeroCross=off
  CompareToConstant2 : simulink/Logic and Bit Operations/Compare To Constant  relop===  const=SYS_NORMAL  OutDataTypeStr=boolean  ZeroCross=off
  Constant2 : Constant  Value=CD_CAN_THROTTLE_FUNCTION
  Logical Operator1 : Logic  AllPortsSameDT=off  OutDataTypeStr=boolean
  Unit Delay1 : UnitDelay  SampleTime=-1
      settings: AttributesFormatString=<tsample=%<SampleTime>>\n<initial=%<X0>>
  Unit Delay2 : UnitDelay  SampleTime=-1
      settings: AttributesFormatString=<tsample=%<SampleTime>>\n<initial=%<X0>>
  cmd_CANThrottle : Outport
  state_CANThrottle : Outport  Port=2
  state_CANThrottleCalDiag : Outport  Port=3
  wires:
  state_System/1 -> CompareToConstant2/1
  CompareToConstant1/1 -> Logical Operator1/1
  Constant2/1 -> CompareToConstant1/1
  CompareToConstant2/1 -> Logical Operator1/2
  Logical Operator1/1 -> CAN_Throttle/Enable
  CAN_Throttle/1(cmd_CANThrottle) -> cmd_CANThrottle/1
  fdbk_CANThrottle/1 -> Unit Delay2/1
  flag_InitEERead/1 -> CAN_Throttle/2(flag_InitEERead)
  CAN_Throttle/2(state_CANThrottle) -> state_CANThrottle/1
  pos_Throttle/1 -> CAN_Throttle/3(pos_Throttle)
  state_Engine/1 -> CAN_Throttle/4(state_Engine)
  state_CommThrottle/1 -> CAN_Throttle/5(state_CommThrottle)
  CAN_Throttle/3(state_CANThrottleCalDiag) -> state_CANThrottleCalDiag/1
  cmd_Throttle/1 -> Unit Delay1/1
  Unit Delay1/1 -> CAN_Throttle/6(cmd_Throttle)
  Unit Delay2/1 -> CAN_Throttle/1(fdbk_CANThrottle)

== system MX_Gateway/MX_Gtwy_Control/System/Controller_State ==
  msgAge_Secondary : Inport
  state_System : Inport  Port=2
  msgAge_ECU : Inport  Port=3
  msgAge_Throttle : Inport  Port=4
  msgAge_StdPanel : Inport  Port=5
  msgAge_JogShuttle : Inport  Port=6
  msgAge_DeluxeG55 : Inport  Port=7
  flag_InitEERead : Inport  Port=8
  msgAge_KeylessEntry : Inport  Port=9
  state_Engine : Inport  Port=10
  state_SwitchedPwrOutput : Inport  Port=11
  state_SwitchedPwrRelay : Inport  Port=12
  error_FuelSec : Inport  Port=13
  state_ECUHold : Inport  Port=14
  Controller_State : Stateflow chart  TreatAsAtomicUnit=on
  state_CommSecondary : Outport
  state_CommECU : Outport  Port=2
  state_CommThrottle : Outport  Port=3
  state_CommStdPanel : Outport  Port=4
  state_CommJogShuttle : Outport  Port=5
  state_CommDeluxeG55 : Outport  Port=6
  state_CommKeylessEntry : Outport  Port=7
  wires:
  state_Engine/1 -> Controller_State/1(state_Engine)
  state_System/1 -> Controller_State/2(state_System)
  state_SwitchedPwrOutput/1 -> Controller_State/3(state_SwitchedPwrOutput)
  state_SwitchedPwrRelay/1 -> Controller_State/4(state_SwitchedPwrRelay)
  msgAge_Secondary/1 -> Controller_State/5(msgAge_Secondary)
  Controller_State/1(state_CommSecondary) -> state_CommSecondary/1
  msgAge_ECU/1 -> Controller_State/6(msgAge_ECU)
  Controller_State/2(state_CommECU) -> state_CommECU/1
  error_FuelSec/1 -> Controller_State/7(error_FuelSec)
  state_ECUHold/1 -> Controller_State/8(state_ECUHold)
  msgAge_Throttle/1 -> Controller_State/9(msgAge_Throttle)
  Controller_State/3(state_CommThrottle) -> state_CommThrottle/1
  Controller_State/4(state_CommStdPanel) -> state_CommStdPanel/1
  Controller_State/5(state_CommJogShuttle) -> state_CommJogShuttle/1
  Controller_State/6(state_CommDeluxeG55) -> state_CommDeluxeG55/1
  msgAge_StdPanel/1 -> Controller_State/10(msgAge_StdPanel)
  msgAge_JogShuttle/1 -> Controller_State/11(msgAge_JogShuttle)
  msgAge_DeluxeG55/1 -> Controller_State/12(msgAge_DeluxeG55)
  flag_InitEERead/1 -> Controller_State/13(flag_InitEERead)
  Controller_State/7(state_CommKeylessEntry) -> state_CommKeylessEntry/1
  msgAge_KeylessEntry/1 -> Controller_State/14(msgAge_KeylessEntry)

== system MX_Gateway/MX_Gtwy_Control/System/Derate ==
  flt_EngineSpeed : Inport  OutDataTypeStr=uint16
  derate_Throttle : Inport  Port=2
  ecu_isocMinRPM : Inport  Port=3
  ecu_isocMaxRPM : Inport  Port=4
  ecu_HP : Inport  Port=5
  state_ECUHold : Inport  Port=6
  state_CommECU : Inport  Port=7
  state_Engine : Inport  Port=8
  Derate : Stateflow chart  TreatAsAtomicUnit=on
  Torque : Lookup  InputValues=MXGTW_ECU_DERATE_RPM  Table=MXGTW_ECU_DERATE_TORQUE  LookUpMeth=Use Input Below  OutDataTypeStr=uint16
      settings: AttributesFormatString=input = %<InputValues>\noutput = %<OutputValues>
  percent_Derate : Outport
  rpm_Derate : Outport  Port=2
  state_EngineSpeedDerate : Outport  Port=3
  state_HPMatch : Outport  Port=4
  torque_Derate : Outport  Port=5
  wires:
  flt_EngineSpeed/1 -> Torque/1, Derate/1(flt_EngineSpeed)  "flt_EngineSpeed"
  Derate/1(percent_Derate) -> percent_Derate/1  "percent_Derate"
  Derate/2(rpm_Derate) -> rpm_Derate/1  "rpm_Derate"
  Derate/3(state_EngineSpeedDerate) -> state_EngineSpeedDerate/1  "state_EngineSpeedDerate"
  ecu_isocMinRPM/1 -> Derate/3(ecu_isocMinRPM)  "ecu_isocMinRPM"
  ecu_isocMaxRPM/1 -> Derate/4(ecu_isocMaxRPM)  "ecu_isocMaxRPM"
  derate_Throttle/1 -> Derate/5(derate_Throttle)  "derate_Throttle"
  Derate/4(state_HPMatch) -> state_HPMatch/1
  state_CommECU/1 -> Derate/6(state_CommECU)
  state_ECUHold/1 -> Derate/7(state_ECUHold)
  ecu_HP/1 -> Derate/8(ecu_HP)
  state_Engine/1 -> Derate/9(state_Engine)
  Torque/1 -> Derate/2(torque_Indicated)
  Derate/5(torque_Derate) -> torque_Derate/1

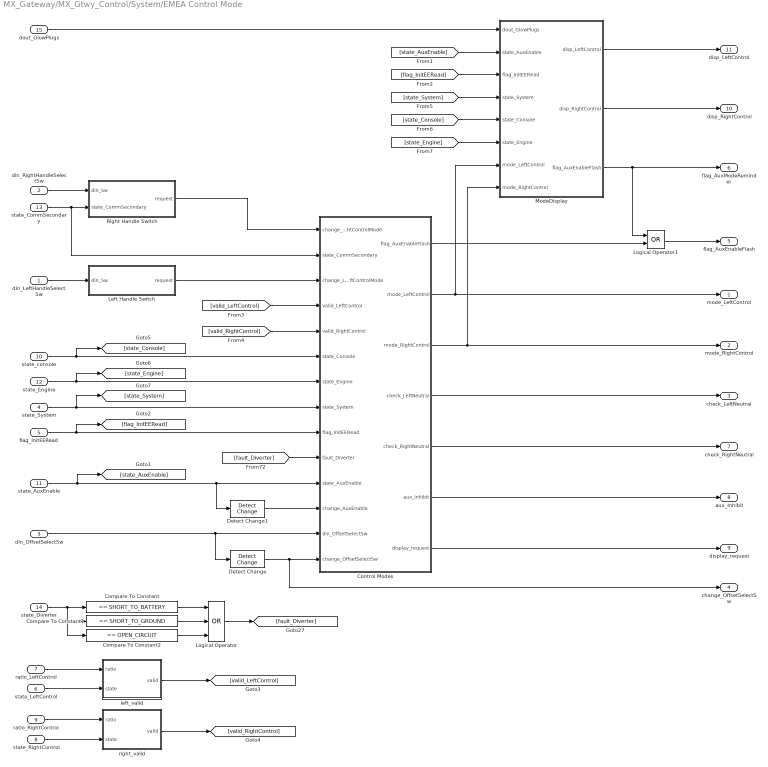
== system MX_Gateway/MX_Gtwy_Control/System/EMEA Control Mode ==
  din_LeftHandleSelectSw : Inport
  din_RightHandleSelectSw : Inport  Port=2
  din_OffsetSelectSw : Inport  Port=3
  state_System : Inport  Port=4
  flag_InitEERead : Inport  Port=5
  state_LeftControl : Inport  Port=6
  ratio_LeftControl : Inport  Port=7
  state_RightControl : Inport  Port=8
  ratio_RightControl : Inport  Port=9
  state_console : Inport  Port=10
  state_AuxEnable : Inport  Port=11
  state_Engine : Inport  Port=12
  state_CommSecondary : Inport  Port=13
  state_Diverter : Inport  Port=14
  dout_GlowPlugs : Inport  Port=15
  Compare To Constant : simulink/Logic and Bit Operations/Compare To Constant  relop===  const=SHORT_TO_BATTERY  OutDataTypeStr=boolean
  Compare To Constant1 : simulink/Logic and Bit Operations/Compare To Constant  relop===  const=SHORT_TO_GROUND  OutDataTypeStr=boolean
  Compare To Constant2 : simulink/Logic and Bit Operations/Compare To Constant  relop===  const=OPEN_CIRCUIT  OutDataTypeStr=boolean
  Control Modes : Stateflow chart  TreatAsAtomicUnit=on
  Detect Change : simulink/Logic and Bit Operations/Detect Change  vinit=0  OutDataTypeStr=boolean
  Detect Change1 : simulink/Logic and Bit Operations/Detect Change  vinit=0  OutDataTypeStr=boolean
  Left Handle Switch : Stateflow chart  TreatAsAtomicUnit=on
  Logical Operator : Logic  Operator=OR  Inputs=3  AllPortsSameDT=off  OutDataTypeStr=boolean
  Logical Operator1 : Logic  Operator=OR  AllPortsSameDT=off  OutDataTypeStr=boolean
  ModeDisplay : Subsystem
  Right Handle Switch : Stateflow chart  TreatAsAtomicUnit=on
  left_valid : Subsystem
  right_valid : Subsystem
  mode_LeftControl : Outport
  mode_RightControl : Outport  Port=2
  check_LeftNeutral : Outport  Port=3
  change_OffsetSelectSw : Outport  Port=4
  flag_AuxEnableFlash : Outport  Port=5
  flag_AuxModeReminder : Outport  Port=6
  check_RightNeutral : Outport  Port=7
  aux_Inhibit : Outport  Port=8
  display_request : Outport  Port=9
  disp_RightControl : Outport  Port=10
  disp_LeftControl : Outport  Port=11
  wires:
  din_LeftHandleSelectSw/1 -> Left Handle Switch/1(din_Sw)
  din_RightHandleSelectSw/1 -> Right Handle Switch/1(din_Sw)
  Left Handle Switch/1(request) -> Control Modes/3(change_LeftControlMode)
  Control Modes/2(mode_LeftControl) -> ModeDisplay/7(mode_LeftControl), mode_LeftControl/1
  Control Modes/3(mode_RightControl) -> ModeDisplay/8(mode_RightControl), mode_RightControl/1
  Right Handle Switch/1(request) -> Control Modes/1(change_RightControlMode)
  state_AuxEnable/1 -> [state_AuxEnable], Detect Change1/1, Control Modes/11(state_AuxEnable)
  din_OffsetSelectSw/1 -> Detect Change/1, Control Modes/13(din_OffsetSelectSw)
  Detect Change/1 -> change_OffsetSelectSw/1, Control Modes/14(change_OffsetSelectSw)
  state_console/1 -> [state_Console], Control Modes/6(state_Console)
  state_Engine/1 -> [state_Engine], Control Modes/7(state_Engine)
  state_System/1 -> [state_System], Control Modes/8(state_System)
  Control Modes/4(check_LeftNeutral) -> check_LeftNeutral/1
  Control Modes/5(check_RightNeutral) -> check_RightNeutral/1
  Detect Change1/1 -> Control Modes/12(change_AuxEnable)
  Control Modes/6(aux_inhibit) -> aux_Inhibit/1
  state_CommSecondary/1 -> Control Modes/2(state_CommSecondary), Right Handle Switch/2(state_CommSecondary)
  state_Diverter/1 -> Compare To Constant2/1, Compare To Constant1/1, Compare To Constant/1
  Compare To Constant/1 -> Logical Operator/1
  Compare To Constant1/1 -> Logical Operator/2
  Compare To Constant2/1 -> Logical Operator/3
  Logical Operator/1 -> [fault_Diverter]
  [fault_Diverter] -> Control Modes/10(fault_Diverter)
  Control Modes/7(display_request) -> display_request/1
  flag_InitEERead/1 -> [flag_InitEERead], Control Modes/9(flag_InitEERead)
  [state_AuxEnable] -> ModeDisplay/2(state_AuxEnable)
  ModeDisplay/3(flag_AuxEnableFlash) -> Logical Operator1/1, flag_AuxModeReminder/1
  Logical Operator1/1 -> flag_AuxEnableFlash/1
  Control Modes/1(flag_AuxEnableFlash) -> Logical Operator1/2
  ModeDisplay/1(disp_LeftControl) -> disp_LeftControl/1
  ModeDisplay/2(disp_RightControl) -> disp_RightControl/1
  [flag_InitEERead] -> ModeDisplay/3(flag_InitEERead)
  dout_GlowPlugs/1 -> ModeDisplay/1(dout_GlowPlugs)
  ratio_LeftControl/1 -> left_valid/1(ratio)
  state_LeftControl/1 -> left_valid/2(state)
  ratio_RightControl/1 -> right_valid/1(ratio)
  state_RightControl/1 -> right_valid/2(state)
  left_valid/1(valid) -> [valid_LeftControl]
  right_valid/1(valid) -> [valid_RightControl]
  [valid_RightControl] -> Control Modes/5(valid_RightControl)
  [valid_LeftControl] -> Control Modes/4(valid_LeftControl)
  [state_System] -> ModeDisplay/4(state_System)
  [state_Console] -> ModeDisplay/5(state_Console)
  [state_Engine] -> ModeDisplay/6(state_Engine)

== system MX_Gateway/MX_Gtwy_Control/System/ElectricAutomation ==
  state_System : Inport
  Bus Creator : BusCreator  Inputs=2
  Bus Selector17 : BusSelector  OutputSignals=CANOpenInMsg.state_Inverter,bus_DigitalInputs.din_RunEnter,bus_AnalogInputs.ain_ThrottlePrimary
  Chart1 : Stateflow chart  TreatAsAtomicUnit=on
  Chart2 : Stateflow chart  TreatAsAtomicUnit=on
  Terminator : Terminator
  targetSpeed : Outport
  wires:
  state_System/1 -> Bus Selector17/1  "bus_Input"
  Bus Selector17/1 -> Chart2/1(tempVar), Chart1/1(state_Inverter)  "<state_Inverter>"
  Chart1/1(cmdInverter) -> Bus Creator/1  "cmdInverter"
  Bus Selector17/2 -> Chart1/2(din_RunEnter)  "<din_RunEnter>"
  Chart1/2(targetSpeed) -> Bus Creator/2  "targetSpeed"
  Bus Creator/1 -> targetSpeed/1  "bus_Inverter"
  Bus Selector17/3 -> Chart1/3(in_Speed)  "<ain_ThrottlePrimary>"
  Chart2/1(testOut) -> Terminator/1  "testOut"

== system MX_Gateway/MX_Gtwy_Control/System/Engine ==
  flt_EngineSpeed : Inport
  state_EngineSpeed : Inport  Port=2  OutDataTypeStr=uint8
  state_ECT : Inport  Port=3  OutDataTypeStr=uint8
  state_EOP : Inport  Port=4  OutDataTypeStr=uint8
  state_HOT : Inport  Port=5  OutDataTypeStr=uint8
  state_EngineStart : Inport  Port=6  OutDataTypeStr=uint8
  state_Key : Inport  Port=7  OutDataTypeStr=uint8
  state_FuelTemp : Inport  Port=8  OutDataTypeStr=uint8
  state_Console : Inport  Port=9  OutDataTypeStr=uint8
  state_System : Inport  Port=10  OutDataTypeStr=uint8
  din_RemoteKey : Inport  Port=11  OutDataTypeStr=uint8
  state_KeylessPanel : Inport  Port=12  OutDataTypeStr=uint8
  can_ST_EStop : Inport  Port=13  OutDataTypeStr=uint8
  can_ACD_EStop : Inport  Port=14  OutDataTypeStr=uint8
  ecu_EngineStarterMode : Inport  Port=15  OutDataTypeStr=uint8
  state_DeluxeG5 : Inport  Port=16  OutDataTypeStr=uint8
  actualHourMeter : Inport  Port=17
  dv_TurboPrime : Inport  Port=18  OutDataTypeStr=uint8
  state_CommECU : Inport  Port=19  OutDataTypeStr=uint8
  state_EngineSpeedDerate : Inport  Port=20  OutDataTypeStr=uint8
  state_CommStdPanel : Inport  Port=21  OutDataTypeStr=uint8
  state_CommDeluxeG55 : Inport  Port=22  OutDataTypeStr=uint8
  Engine : Stateflow chart  TreatAsAtomicUnit=on
  state_Engine : Outport  OutDataTypeStr=uint8
  state_TurboPrime : Outport  Port=2  OutDataTypeStr=uint8
  wires:
  Engine/1(state_Engine) -> state_Engine/1  "state_Engine"
  flt_EngineSpeed/1 -> Engine/1(flt_EngineSpeed)  "flt_EngineSpeed"
  state_EngineSpeed/1 -> Engine/2(state_EngineSpeed)  "state_EngineSpeed"
  state_ECT/1 -> Engine/3(state_ECT)  "state_ECT"
  state_EOP/1 -> Engine/4(state_EOP)  "state_EOP"
  state_HOT/1 -> Engine/5(state_HOT)  "state_HOT"
  state_EngineStart/1 -> Engine/6(state_EngineStart)  "state_EngineStart"
  state_Key/1 -> Engine/7(state_Key)  "state_Key"
  state_System/1 -> Engine/8(state_System)  "state_System"
  din_RemoteKey/1 -> Engine/9(din_RemoteKey)  "din_RemoteKey"
  state_KeylessPanel/1 -> Engine/10(state_KeylessPanel)  "state_KeylessPanel"
  state_CommECU/1 -> Engine/11(state_CommECU)  "state_CommECU"
  can_ST_EStop/1 -> Engine/12(can_ST_EStop)  "can_ST_EStop"
  ecu_EngineStarterMode/1 -> Engine/14(ecu_EngineStarterMode)  "ecu_GlowPlug"
  state_EngineSpeedDerate/1 -> Engine/15(state_EngineSpeedDerate)
  can_ACD_EStop/1 -> Engine/13(can_ACD_EStop)
  state_FuelTemp/1 -> Engine/16(state_FuelTemp)
  state_DeluxeG5/1 -> Engine/18(state_DeluxeG5)
  state_CommDeluxeG55/1 -> Engine/19(state_CommDeluxeG55)
  state_CommStdPanel/1 -> Engine/17(state_CommStdPanel)
  state_Console/1 -> Engine/20(state_Console)
  actualHourMeter/1 -> Engine/21(actualHourMeter)
  dv_TurboPrime/1 -> Engine/22(dv_TurboPrime)
  Engine/2(state_TurboPrime) -> state_TurboPrime/1
